simulink model "ofdm_txrx_supermimo_coded"  (Simulink 7.1)
settings: Solver=VariableStepDiscrete  SolverMode=SingleTasking  StartTime=0.0  StopTime=2*simOnly_simLength  RelTol=1e-3
PostLoadFcn:
  | ofdm_rx_supermimo_init
InitFcn:
  | ofdm_rx_supermimo_init
StartFcn:
  | ofdm_rx_supermimo_init
values from neighbouring files (symbols this model uses):
  Action0_Config = 0* 31*2^18 + 0*2^24 + PHY_AUTORESPONSE_REQ_GOODHDR + PHY_AUTORESPONSE_REQ_GOODPKT + PHY_AUTORESPONSE_REQ_FLAGA    [ofdm_tx_supermimo_init.m]
  Action1_Config = 0* 62*2^18 + PHY_AUTORESPONSE_REQ_GOODHDR + PHY_AUTORESPONSE_REQ_GOODPKT    [ofdm_tx_supermimo_init.m]
  Action2_Config = 0    [ofdm_tx_supermimo_init.m]
  Action3_Config = 0    [ofdm_tx_supermimo_init.m]
  Action4_Config = 0    [ofdm_tx_supermimo_init.m]
  Action5_Config = 0    [ofdm_tx_supermimo_init.m]
  ActionID_AFTransmit = 31    [ofdm_tx_supermimo_init.m]
  ActionID_Disabled = 0    [ofdm_tx_supermimo_init.m]
  ActionID_SetFlagA = 62    [ofdm_tx_supermimo_init.m]
  ActionID_SetFlagB = 61    [ofdm_tx_supermimo_init.m]
  BER_RAM_init_size = 2048    [ofdm_tx_supermimo_init.m]
  channel_h = [1 0; 1 0] * 0.3    [ofdm_tx_supermimo_init.m]
  CPLength = 16    [ofdm_tx_supermimo_init.m]
  FEC_Config = fec_coding_en*1 + fec_soft_dec*2 + fec_qpsk_scl*2^4 + fec_16qam_scl*2^8    (= 4195)    [ofdm_tx_supermimo_init.m]
  longCorr_coef_i = [-4*real(LTS) 0]    [ofdm_rx_supermimo_init.m]
  longCorr_coef_q = [4*imag(LTS) 0]    [ofdm_rx_supermimo_init.m]
  Match0_Config = 0    [ofdm_tx_supermimo_init.m]
  Match1_Config = 0    [ofdm_tx_supermimo_init.m]
  Match2_Config = 0    [ofdm_tx_supermimo_init.m]
  Match3_Config = 0    [ofdm_tx_supermimo_init.m]
  Match4_Config = 0    [ofdm_tx_supermimo_init.m]
  Match5_Config = 0    [ofdm_tx_supermimo_init.m]
  Match6_Config = 0    [ofdm_tx_supermimo_init.m]
  Match7_Config = 0    [ofdm_tx_supermimo_init.m]
  max_CP_length = 16    [ofdm_tx_supermimo_init.m]
  max_num_baseRateSymbols = 31    [ofdm_tx_supermimo_init.m]
  max_num_subcarriers = 64    [ofdm_tx_supermimo_init.m]
  max_num_trainingSymbols = 15    [ofdm_tx_supermimo_init.m]
  max_numBytes = 16384    [ofdm_tx_supermimo_init.m]
  max_OFDM_symbols = 1023    [ofdm_tx_supermimo_init.m]
  modConstellation_qam16 = .75*[1 3 -1 -3]./3    [ofdm_tx_supermimo_init.m]
  modConstellation_qam64 = 0.875*[3 1 5 7 -3 -1 -5 -7]./7    [ofdm_tx_supermimo_init.m]
  modConstellation_qpsk = [1 -1]./sqrt(2)    [ofdm_tx_supermimo_init.m]
  numBaseRateSymbols = numBaseRateSymbols *2    [ofdm_tx_supermimo_init.m]
  numSubcarriers = 64    [ofdm_tx_supermimo_init.m]
  numTrainingSymbols = 2    [ofdm_tx_supermimo_init.m]
  pilot_indicies = 7 + ( (64-21) * 2^8) + ( (64-7) * 2^16) + (21 * 2^24)    (= 356068103)    [ofdm_tx_supermimo_init.m]
  pilotValues = hex2dec('A57D') + (2^16 * hex2dec('5A82'))    [ofdm_tx_supermimo_init.m]
  pktByteNums = numHeaderBytes +  				byteIndex_numPayloadBytes(1)*2^8 +  				byteIndex_numPayloadBytes(2)*2^16 +  				byteIndex_simpleDynModMasks * 2^24    [ofdm_rx_supermimo_init.m]
  PktDet_Delay = 58+32 +      Symbol_Timing_Offset*2^7 +      0*2^16 +      (117* 2^24)    (= 1962935642)    [ofdm_rx_supermimo_init.m]
  pktTiming_count_payloadStart = pktTiming_count_lsStart + pktTiming_ls_num*pktTiming_ls_length    [ofdm_rx_supermimo_init.m]
  preamble = preamble_scale*[shortsyms_10 longsyms_2]    [ofdm_tx_supermimo_init.m]
  preamble_ROM = preamble_I_ROM + preamble_Q_ROM*2^16    [ofdm_tx_supermimo_init.m]
  RAM_init_size = max_numBytes    (= 16384)    [ofdm_tx_supermimo_init.m]
  reg_AF_Tx_Blanking = (320+80) + ( (320+80+79)*2^16)    (= 31392144)    [ofdm_rx_supermimo_init.m]
  reg_AF_Tx_Scaling = 2944    [ofdm_rx_supermimo_init.m]
  reg_Interrupt_PktBuf_Control = reg_InterruptControl +  	1 * 2^16 +  	0 * 2^24    (= 65536)    [ofdm_rx_supermimo_init.m]
  reg_PilotCalcParams = (4 * 2^16) + (minPilotChanMag)    (= 262184.96)    [ofdm_rx_supermimo_init.m]
  regRx_chanEst_minMag = ((0.5)*2^15) + ((0.5 * 2^15)*2^16)    (= 1073758208)    [ofdm_rx_supermimo_init.m]
  regRx_coarseCalcCorrection = round((1.3e-3 - 1.2982e-3)*2^32)    [ofdm_rx_supermimo_init.m]
  regRx_pilotCalcCorrection = round(1.00115 * 2^31)    [ofdm_rx_supermimo_init.m]
  regRx_preCFOoptions = 0 * 2^0 +  	1 * 2^1 +  	0    (= 2)    [ofdm_rx_supermimo_init.m]
  rx_controlBits = 1 * 2  		 + 1 * 4  		 + 0 * 8  		 + 1 * 16 * rx_SISO_Mode  		 + 1 * 32  		 + 1 * 64  		 + 1 * 128  		 + 1 * 256  		 + 1 * 512  		 + 1 * 1024  		 + 1 * 4096  		 + 0 * 16384  		 + 0 * 32768  		 + 1 * 65536  		 + 0 * 131072  		 + 0 * 262144  		 + 1 * 524288  		 + 1 * 1048576 * tx_Alamouti_Mode  		 + 0 * 2097152           + 1 * 33554432  		 + 0 * 4194304  		 + 0 * 8388608  		 + 0 * 16777216  		 + 1 *  ...(+49 chars)    [ofdm_rx_supermimo_init.m]
  Rx_PktDet_LongCorr_Params = LongCorrCounterLoad + 0*2^8 + 0*2^16 + 255*2^24    (= 4278190325)    [ofdm_rx_supermimo_init.m]
  Rx_PktDet_LongCorrThresholds = 8000    [ofdm_rx_supermimo_init.m]
  rx_postEq_scaling = round(rxScaling*2^11) * (1 + 2^16)    [ofdm_rx_supermimo_init.m]
  RxDataScrambling_Seq = [40 198 78 63 82 173 102 245 48 111 172 115 147 230 216 93 72 65 62 2 205 242 122 90 128 83 105 97 73 10 5 252]    [ofdm_tx_supermimo_init.m]
  RxReg_FixedPktLen = 0    [ofdm_rx_supermimo_init.m]
  rxReg_pktDetCorr = 2^00 * (floor(0.7*128)) +  	2^08 * (300) +  	0    [ofdm_rx_supermimo_init.m]
  shortSymbol_time = shortSymbol_time(1:16).'    [ofdm_tx_supermimo_init.m]
  simOnly_numSamples = length(preamble)+( (numSubcarriers+CPLength)*(numTrainingSymbols + numBaseRateSymbols + numFullRateSymbols/2) )    [ofdm_tx_supermimo_init.m]
  train = [train train]    [ofdm_tx_supermimo_init.m]
  tx_controlBits = (antB_preambleShift * 2^4) + (2^1 * tx_Alamouti_Mode) +  	(2^0 * tx_SISO_Mode) + 4*0 + 8*1 + 0*256 + 512*0 + 1024*0 +  	0*hex2dec('1000') + 0*hex2dec('2000') + 0*hex2dec('4000') +  	1*hex2dec('8000') + 1*hex2dec('10000') + hex2dec('20000') +  	0*hex2dec('100000')    [ofdm_tx_supermimo_init.m]
  tx_delays = (50*2^0) + (0*2^8) + (30*2^12)    (= 122930)    [ofdm_tx_supermimo_init.m]
  tx_scaling = (3072) + (12288 * 2^16)    (= 805309440)    [ofdm_tx_supermimo_init.m]
  TxDataScrambling_Seq = [40 198 78 63 82 173 102 245 48 111 172 115 147 230 216 93 72 65 62 2 205 242 122 90 128 83 105 97 73 10 5 252]    [ofdm_tx_supermimo_init.m]
  txReg_symbolCounts = (2^8 *numBaseRateSymbols ) + numTrainingSymbols    [ofdm_tx_supermimo_init.m]
  TxRx_FFTScaling = (3 * 2^0) +  	(2 * 2^2) +  	(1 * 2^4) +  	(1 * 2^6) +  	(1 * 2^8) +  	(0 * 2^10)    (= 347)    [ofdm_tx_supermimo_init.m]
undefined symbols (not defined in the file or in its folder): AntA, AntB, BaseRate, Down, gcbh, numBuffs, numBytes, Up, xlBlockLoadCallback, xlGetSimulinkPeriod, xlipgetpartsetting
libraries: xbsIndex_r4 x6000, simulink x4, dspsigops x4, commchan3 x2, dspsrcs4 x2, commrflib2 x2
(part 7 of 63)

== system ofdm_txrx_supermimo_coded/OFDM Rx MIMO/Coarse Freq Correction ==
  Reset : Inport  OutDataType=sfix(16)  OutScaling=2^0
  PktDet : Inport  Port=2  OutDataType=sfix(16)  OutScaling=2^0
  AntA I : Inport  Port=3  OutDataType=sfix(16)  OutScaling=2^0
  AntA Q : Inport  Port=4  OutDataType=sfix(16)  OutScaling=2^0
  AntB I : Inport  Port=5  OutDataType=sfix(16)  OutScaling=2^0
  AntB Q : Inport  Port=6  OutDataType=sfix(16)  OutScaling=2^0
  A*B : Subsystem
  A*B1 : Subsystem
  Bus Creator1 : BusCreator  Inputs=2
  CFO : Scope  NumInputPorts=5
      settings: TimeRange=14608  YMin=-0.25~-0.25~-1~-1~0  YMax=0.2~0.25~1~1~1  SaveName=ScopeData26
  Coarse CFO Calc : Subsystem
  Complex to Magnitude-Angle : ComplexToMagnitudeAngle
  Complex to Real-Imag : ComplexToRealImag
  Convert{,1..3} (4 alike) : xbsIndex_r4/Convert  arith_type="Signed  (2's comp)"  n_bits=16  bin_pt=15  quantization=Truncate  overflow=Flag as error  latency=0  dbl_ovrd=off  pipeline=off  block_type=convert
      settings: xl_area=[0 0 0 0 0 0 0]  block_version=VER_STRING_GOES_HERE
  DDS : Subsystem
  Gateway Out{,1..3} (4 alike) : xbsIndex_r4/Gateway Out  hdl_port=off  timing_constraint=None  locs_specified=off  LOCs={}  block_type=gatewayout
      settings: infoedit="Gateway out block.  Converts Xilinx fixed point inputs into ouputs of type Simulink integer, double, or fixed point.<P><P>Hardware notes:  In hardware these blocks become top level output ports or are discarded, depending on how they are configured."  xl_area=[0 0 0 0 0 0 0]  block_version=VER_STRING_GOES_HERE
  Mux2 : Mux  Inputs=2
  PreSpin CFO Sel : Subsystem
  Product : Product  OutDataType=sfix(16)  OutScaling=2^0  RndMeth=Zero
  Real-Imag to Complex : RealImagToComplex
  "AntA I " : Outport  OutDataType=sfix(16)  OutScaling=2^0
  "AntA Q " : Outport  Port=2  OutDataType=sfix(16)  OutScaling=2^0
  "AntB I " : Outport  Port=3  OutDataType=sfix(16)  OutScaling=2^0
  "AntB Q " : Outport  Port=4  OutDataType=sfix(16)  OutScaling=2^0
  tag scope: CFO_PhaseInc=global
  wires:
  A*B/1(Re) -> Convert/1
  A*B/2(Im1) -> Convert1/1
  A*B1/1(Re) -> Convert2/1
  A*B1/2(Im1) -> Convert3/1
  DDS/1(Q) -> Gateway Out/1, A*B1/2(Im[B]), A*B/3(Im[B])
  DDS/2(I) -> Gateway Out1/1, A*B1/1(Re[B]), A*B/4(Re[B])
  Gateway Out1/1 -> Mux2/1, Real-Imag to Complex/1
  Gateway Out/1 -> Mux2/2, Real-Imag to Complex/2
  Mux2/1 -> CFO/3
  Bus Creator1/1 -> CFO/4
  [freqOffset] -> Complex to Real-Imag/1, Product/2
  Complex to Real-Imag/1 -> Bus Creator1/1
  Complex to Real-Imag/2 -> Bus Creator1/2
  Gateway Out2/1 -> CFO/2
  Gateway Out3/1 -> CFO/1
  AntB I/1 -> A*B1/3(Re[A])
  AntB Q/1 -> A*B1/4(Im[A])
  AntA I/1 -> A*B/1(Re[A]), Coarse CFO Calc/1(I In)
  AntA Q/1 -> A*B/2(Im[A]), Coarse CFO Calc/2(Q In)
  Convert/1 -> "AntA I "/1
  Convert1/1 -> "AntA Q "/1
  Real-Imag to Complex/1 -> Product/1
  Product/1 -> Complex to Magnitude-Angle/1
  Complex to Magnitude-Angle/2 -> CFO/5
  Convert2/1 -> "AntB I "/1
  Convert3/1 -> "AntB Q "/1
  Reset/1 -> Coarse CFO Calc/4(Reset), DDS/3(Rst), PreSpin CFO Sel/1(Rst)
  PktDet/1 -> Gateway Out2/1, Coarse CFO Calc/3(RxPkt Active)
  Coarse CFO Calc/1(Phase Inc) -> Gateway Out3/1, [CFO_PhaseInc], DDS/2(Rx_CoarseEst)
  PreSpin CFO Sel/1(Tx_PhaseInc) -> DDS/1(Tx_PhaseInc)

== system ofdm_txrx_supermimo_coded/OFDM Rx MIMO/Equalizer & Packetizer ==
  Payload : Inport  OutDataType=sfix(16)  OutScaling=2^0
  Y_I : Inport  Port=2  OutDataType=sfix(16)  OutScaling=2^0
  Y_Q : Inport  Port=3  OutDataType=sfix(16)  OutScaling=2^0
  H-I : Inport  Port=4  OutDataType=sfix(16)  OutScaling=2^0
  H-Q : Inport  Port=5  OutDataType=sfix(16)  OutScaling=2^0
  SubCar Index : Inport  Port=6  OutDataType=sfix(16)  OutScaling=2^0
  Sym Index : Inport  Port=7  OutDataType=sfix(16)  OutScaling=2^0
  Vin : Inport  Port=8  OutDataType=sfix(16)  OutScaling=2^0
  Phases I : Inport  Port=9  OutDataType=sfix(16)  OutScaling=2^0
  Phases Q : Inport  Port=10  OutDataType=sfix(16)  OutScaling=2^0
  Convert4 : xbsIndex_r4/Convert  arith_type="Signed  (2's comp)"  n_bits=14  bin_pt=13  quantization=Truncate  overflow=Wrap  latency=0  dbl_ovrd=off  pipeline=off  block_type=convert
      settings: xl_area=[0, 0, 0, 0, 0, 0, 0]  block_version=VER_STRING_GOES_HERE
  Convert5 : xbsIndex_r4/Convert  arith_type="Signed  (2's comp)"  n_bits=14  bin_pt=13  quantization=Truncate  overflow=Wrap  latency=0  dbl_ovrd=off  pipeline=off  block_type=convert
      settings: xl_area=[0, 0, 0, 0, 0, 0, 0]  block_version=VER_STRING_GOES_HERE
  Eq : Scope  NumInputPorts=7
      settings: YMin=-0.5~-0.5~0~0~15~0.95~-1  YMax=0.5~0.5~70~1~20~1.05~1  SaveName=ScopeData14
  Equalizer : Subsystem
  Packet_Constructor : Subsystem
  Real-Imag to Complex : RealImagToComplex
  Rx_debug_EQ_I : xbsIndex_r4/Gateway Out  hdl_port=on  timing_constraint=None  locs_specified=off  LOCs={}  block_type=gatewayout
      settings: infoedit="Gateway out block.  Converts Xilinx fixed point inputs into ouputs of type Simulink integer, double, or fixed point.<P><P>Hardware notes:  In hardware these blocks become top level output ports or are discarded, depending on how they are configured."  xl_area=[0 0 0 0 1 0 0]  block_version=VER_STRING_GOES_HERE
  Rx_debug_EQ_Q : xbsIndex_r4/Gateway Out  hdl_port=on  timing_constraint=None  locs_specified=off  LOCs={}  block_type=gatewayout
      settings: infoedit="Gateway out block.  Converts Xilinx fixed point inputs into ouputs of type Simulink integer, double, or fixed point.<P><P>Hardware notes:  In hardware these blocks become top level output ports or are discarded, depending on how they are configured."  xl_area=[0 0 0 0 1 0 0]  block_version=VER_STRING_GOES_HERE
  Scaling : Subsystem
  Up Sample1 : xbsIndex_r4/Up Sample  sample_ratio=2  copy_samples=on  dbl_ovrd=off  block_type=usamp
      settings: infoedit="Up samples input data.  Inserted values  can be zeros or copies of the most recent input sample.<P><P>Hardware notes: No hardware is needed if inserted values are copies of the input sample; otherwise, a mux and single bit flip-flop are used."  block_version=8.2.02
  Up Sample2 : xbsIndex_r4/Up Sample  sample_ratio=2  copy_samples=on  dbl_ovrd=off  block_type=usamp
      settings: infoedit="Up samples input data.  Inserted values  can be zeros or copies of the most recent input sample.<P><P>Hardware notes: No hardware is needed if inserted values are copies of the input sample; otherwise, a mux and single bit flip-flop are used."  block_version=8.2.02
  Zero-Order Hold : ZeroOrderHold
  Zero-Order Hold1 : ZeroOrderHold
  done{1..6} (6 alike) : xbsIndex_r4/Gateway Out  hdl_port=off  timing_constraint=None  locs_specified=off  LOCs={}  block_type=gatewayout
      settings: infoedit="Gateway out block.  Converts Xilinx fixed point inputs into ouputs of type Simulink integer, double, or fixed point.<P><P>Hardware notes:  In hardware these blocks become top level output ports or are discarded, depending on how they are configured."  xl_area=[0 0 0 0 1 0 0]  block_version=VER_STRING_GOES_HERE
  posedge : Subsystem
  done : Outport  OutDataType=sfix(16)  OutScaling=2^0
  wires:
  SubCar Index/1 -> Equalizer/9(SubCar Index)
  Sym Index/1 -> Equalizer/8(Sym Index)
  Scaling/1(I) -> Packet_Constructor/1(I), Convert4/1
  Scaling/2(Q) -> Packet_Constructor/2(Q), Convert5/1
  Scaling/3(" Xk_index") -> Packet_Constructor/3(Xk_index)
  Scaling/4(Vout) -> Packet_Constructor/4(Vin)
  Scaling/5(" Sym Index") -> Packet_Constructor/5(Sym Index)
  Packet_Constructor/1(done) -> done/1
  H-I/1 -> Equalizer/5(H-I)
  H-Q/1 -> Equalizer/6(H-Q)
  Vin/1 -> Equalizer/7(Vin)
  Y_I/1 -> Equalizer/3(Y_I)
  Y_Q/1 -> Equalizer/4(Y_Q)
  Payload/1 -> posedge/1(D), done6/1
  posedge/1(Q) -> Packet_Constructor/6(Start)
  Equalizer/1(I-eq) -> Scaling/1(Eq-I), done4/1
  Equalizer/2(Q-eq) -> Scaling/2(Eq-Q), done5/1
  Equalizer/3(" Xk_index") -> Scaling/3(Xk_index), done1/1
  Equalizer/4(Vout) -> Scaling/4(Vin), done2/1
  Equalizer/5(" Sym Index") -> Scaling/5(Sym Index), done3/1
  Up Sample2/1 -> Rx_debug_EQ_Q/1
  Up Sample1/1 -> Rx_debug_EQ_I/1
  Convert5/1 -> Up Sample2/1
  Convert4/1 -> Up Sample1/1
  Phases I/1 -> Equalizer/1(Phases I)
  Phases Q/1 -> Equalizer/2(Phases Q)
  Rx_debug_EQ_I/1 -> Zero-Order Hold/1
  Rx_debug_EQ_Q/1 -> Zero-Order Hold1/1
  Real-Imag to Complex/1 -> ?
  Zero-Order Hold/1 -> Real-Imag to Complex/1
  Zero-Order Hold1/1 -> Real-Imag to Complex/2
  done4/1 -> Eq/1
  done5/1 -> Eq/2
  done1/1 -> Eq/3
  done2/1 -> Eq/4
  done3/1 -> Eq/5
  done6/1 -> Eq/6

== system ofdm_txrx_supermimo_coded/OFDM Rx MIMO/FFT & Chan Est ==
  note: Payload->WE latency was 1 - why?
  Payload : Inport  OutDataType=sfix(16)  OutScaling=2^0
  AntA I : Inport  Port=2  OutDataType=sfix(16)  OutScaling=2^0
  AntA Q : Inport  Port=3  OutDataType=sfix(16)  OutScaling=2^0
  AntB I : Inport  Port=4  OutDataType=sfix(16)  OutScaling=2^0
  AntB Q : Inport  Port=5  OutDataType=sfix(16)  OutScaling=2^0
  Addr Gen1 : Subsystem
  Addr_Generator : Subsystem
  Assert : xbsIndex_r4/Assert  Orientation=left  assert_type=off  type_source=Explicitly  arith_type="Signed  (2's comp)"  n_bits=16  bin_pt=14  assert_rate=on  rate_source=Explicitly  period=1  output_port=on  block_type=assert
      settings: infoedit=Asserts a user-defined sample rate and/or type on a signal.<P><P>Hardware notes: In hardware this block costs nothing.  block_version=VER_STRING_GOES_HERE
  Channel Estimation & Pilot Processing : Subsystem
  Delay{1..4} (4 alike) : xbsIndex_r4/Delay  en=off  latency=1  dbl_ovrd=off  reg_retiming=off  block_type=delay
      settings: infoedit=Hardware notes: A delay line is a chain, each link of which is an SRL16 followed by a flip-flop. If register retiming is enabled, the delay line is a chain of flip-flops.  block_version=VER_STRING_GOES_HERE
  Down Sample : xbsIndex_r4/Down Sample  Orientation=left  sample_ratio=2  sample_phase=First Value of Frame  en=off  latency=0  dbl_ovrd=off  block_type=dsamp
      settings: block_version=VER_STRING_GOES_HERE
  FFT : Subsystem
  Input Buffers : Subsystem
  Inverter2 : xbsIndex_r4/Inverter  en=off  latency=0  dbl_ovrd=off  block_type=inv
      settings: block_version=VER_STRING_GOES_HERE
  Output Buffers : Subsystem
  Symbol Counter : Subsystem
  Y_I : Outport  OutDataType=sfix(16)  OutScaling=2^0
  Y_Q : Outport  Port=2  OutDataType=sfix(16)  OutScaling=2^0
  H-I : Outport  Port=3  OutDataType=sfix(16)  OutScaling=2^0
  H-Q : Outport  Port=4  OutDataType=sfix(16)  OutScaling=2^0
  SubCar Index : Outport  Port=5  OutDataType=sfix(16)  OutScaling=2^0
  Sym Index : Outport  Port=6  OutDataType=sfix(16)  OutScaling=2^0
  Vout : Outport  Port=7  OutDataType=sfix(16)  OutScaling=2^0
  Phase I : Outport  Port=8  OutDataType=sfix(16)  OutScaling=2^0
  Phase Q : Outport  Port=9  OutDataType=sfix(16)  OutScaling=2^0
  tag scope: FFT_I=global
  wires:
  Delay1/1 -> Input Buffers/5(We)
  FFT/5(Done) -> Symbol Counter/1(FFT Done), Addr Gen1/1(FFT Done)
  Addr_Generator/3(Ant Sel) -> Input Buffers/8(Ant Sel)
  Assert/1 -> Addr_Generator/2(FFT Xn_index)
  AntB Q/1 -> Input Buffers/4(AntB Q)
  AntB I/1 -> Input Buffers/3(AntB I)
  AntA Q/1 -> Input Buffers/2(AntA Q)
  AntA I/1 -> Input Buffers/1(AntA I)
  Payload/1 -> Delay1/1, Addr_Generator/1(Payload), Symbol Counter/2(Payload), Addr Gen1/2(Payload), Inverter2/1
  Addr_Generator/2(Read Addr) -> Input Buffers/7(Rd Addr)
  Addr_Generator/5(FFT Start) -> FFT/3(Start)
  Input Buffers/2(" Q") -> FFT/2(xn_Q)
  Input Buffers/1(" I") -> FFT/1(xn_I)
  Addr_Generator/1(Write Addr) -> Input Buffers/6(Wr Addr)
  Delay4/1 -> SubCar Index/1
  Delay2/1 -> Vout/1
  Output Buffers/2(" Q") -> Y_Q/1
  Channel Estimation & Pilot Processing/2(H-Q) -> H-Q/1
  Channel Estimation & Pilot Processing/1(H-I) -> H-I/1
  Output Buffers/1(" I") -> Y_I/1
  Channel Estimation & Pilot Processing/5(Est Vout) -> Down Sample/1
  Symbol Counter/1(Sym Index) -> Channel Estimation & Pilot Processing/5(Sym Index), Output Buffers/5(SymIndex)
  FFT/4(Vout) -> Channel Estimation & Pilot Processing/4(Vin), Output Buffers/4(FFT Vout)
  FFT/3(xk_Index) -> Channel Estimation & Pilot Processing/3(Xk Index), Output Buffers/3(Wr Addr)
  FFT/2(xk_Q) -> Output Buffers/2(Q), Channel Estimation & Pilot Processing/2(Q)
  FFT/1(xk_I) -> Channel Estimation & Pilot Processing/1(I), Output Buffers/1(I), [FFT_I]
  FFT/6(xn_Index) -> Assert/1
  Down Sample/1 -> Addr Gen1/3(NotTraining)
  Addr Gen1/2(Rd Addr) -> Output Buffers/6(Rd Addr), Channel Estimation & Pilot Processing/6(Est Index), Delay4/1
  Addr Gen1/3(Vout) -> Delay2/1
  Delay3/1 -> Sym Index/1
  Addr Gen1/1(SymIndex_out) -> Delay3/1
  Addr_Generator/4(Wr Slot) -> Input Buffers/9(Wr Slot)
  Channel Estimation & Pilot Processing/3(Phase I) -> Phase I/1
  Channel Estimation & Pilot Processing/4(Phase Q) -> Phase Q/1
  Inverter2/1 -> Channel Estimation & Pilot Processing/7(Rst)

== system ofdm_txrx_supermimo_coded/OFDM Rx MIMO/Long Corr HP ==
  note: This block was originally designed by Dr. Chris Dick (Cheif DSP Scientist at Xilinx)
      | It's been modified since to run at 4x (instead of 5x, as in the original OFDM designs)
      |
      | This block runs a 64-point 1-bit by 1-bit correlation per clock cycle. Using 1-bit per
      | operand allows just add/sub operations instead of actual multiplicaitons,
      | which would be hugely expensive. This degrades performance a little,
      | but the LTS have good enough autocorrelation properties that there is still
      | plenth of margin using this scheme.
  I In : Inport  OutDataType=sfix(16)  OutScaling=2^0
  Q In : Inport  Port=2  OutDataType=sfix(16)  OutScaling=2^0
  AddSub : xbsIndex_r4/AddSub  mode=Subtraction  use_carryin=off  use_carryout=off  en=off  latency=0  precision=Full  arith_type=Unsigned  n_bits=8  bin_pt=2  quantization=Truncate  overflow=Wrap  dbl_ovrd=off  use_behavioral_HDL=on  pipelined=off  use_rpm=off  hw_selection=Fabric  block_type=addsub
      settings: xl_area=[5 0 0 8 0 0 0]  block_version=VER_STRING_GOES_HERE
  AddSub1 : xbsIndex_r4/AddSub  mode=Addition  use_carryin=off  use_carryout=off  en=off  latency=0  precision=Full  arith_type=Unsigned  n_bits=8  bin_pt=2  quantization=Truncate  overflow=Wrap  dbl_ovrd=off  use_behavioral_HDL=on  pipelined=off  use_rpm=off  hw_selection=Fabric  block_type=addsub
      settings: xl_area=[5 0 0 8 0 0 0]  block_version=VER_STRING_GOES_HERE
  AddSub2 : xbsIndex_r4/AddSub  mode=Addition  use_carryin=off  use_carryout=off  en=off  latency=0  precision=Full  arith_type=Unsigned  n_bits=8  bin_pt=2  quantization=Truncate  overflow=Wrap  dbl_ovrd=off  use_behavioral_HDL=on  pipelined=off  use_rpm=off  hw_selection=Fabric  block_type=addsub
      settings: xl_area=[10 0 0 18 0 0 0]  block_version=VER_STRING_GOES_HERE
  C1 : Subsystem  mask{ns=0, N=4, h=longCorr_coef_i, Ts_In=4}
      mask prompts: ns "Num sub-correlator"; N "Coefficients/correlator"; h "Coefficients"; Ts_In "Input sample period"
  C2 : Subsystem  mask{ns=0, N=4, h=longCorr_coef_q, Ts_In=4}
      mask prompts: ns "Num sub-correlator"; N "Coefficients/correlator"; h "Coefficients"; Ts_In "Input sample period"
  C3 : Subsystem  mask{ns=0, N=4, h=longCorr_coef_i, Ts_In=4}
      mask prompts: ns "Num sub-correlator"; N "Coefficients/correlator"; h "Coefficients"; Ts_In "Input sample period"
  C4 : Subsystem  mask{ns=0, N=4, h=longCorr_coef_q, Ts_In=4}
      mask prompts: ns "Num sub-correlator"; N "Coefficients/correlator"; h "Coefficients"; Ts_In "Input sample period"
  Mult : xbsIndex_r4/Mult  precision=Full  arith_type="Signed  (2's comp)"  n_bits=8  bin_pt=2  quantization=Truncate  overflow=Wrap  en=off  latency=1  dbl_ovrd=off  use_behavioral_HDL=off  use_embedded=on  opt=Speed  optimum_pipeline=off  pipeline=on  use_rpm=off  placement_style=Rectangular shape  block_type=mult
      settings: infoedit=Hardware notes: To use the internal pipeline stage of the dedicated multiplier you must select 'Pipeline for maximum performance'.  xl_area=[60 18 0 106 0 0 0]  block_version=VER_STRING_GOES_HERE
  Mult1 : xbsIndex_r4/Mult  precision=Full  arith_type="Signed  (2's comp)"  n_bits=8  bin_pt=2  quantization=Truncate  overflow=Wrap  en=off  latency=1  dbl_ovrd=off  use_behavioral_HDL=off  use_embedded=on  opt=Speed  optimum_pipeline=off  pipeline=on  use_rpm=off  placement_style=Rectangular shape  block_type=mult
      settings: infoedit=Hardware notes: To use the internal pipeline stage of the dedicated multiplier you must select 'Pipeline for maximum performance'.  xl_area=[60 18 0 106 0 0 0]  block_version=VER_STRING_GOES_HERE
  Slice : xbsIndex_r4/Slice  nbits=1  boolean_output=off  mode=Upper Bit Location + Width  bit1=0  base1=MSB of Input  bit0=0  base0=LSB of Input  dbl_ovrd=off  block_type=slice
      settings: infoedit="Extracts a given range of bits from each input sample and presents it at the output.  The output type is ordinarily unsigned with binary point at zero, but can be Boolean when the slice is one bit wide.<P><P>Hardware notes: In hardware this block costs nothing."  block_version=10.1.3
  Slice1 : xbsIndex_r4/Slice  nbits=1  boolean_output=off  mode=Upper Bit Location + Width  bit1=0  base1=MSB of Input  bit0=0  base0=LSB of Input  dbl_ovrd=off  block_type=slice
      settings: infoedit="Extracts a given range of bits from each input sample and presents it at the output.  The output type is ordinarily unsigned with binary point at zero, but can be Boolean when the slice is one bit wide.<P><P>Hardware notes: In hardware this block costs nothing."  block_version=10.1.3
  y : Outport  OutDataType=sfix(16)  OutScaling=2^0
  tag scope: Long_Corr=global
  wires:
  Slice1/1 -> C2/1(x), C3/1(x)
  Slice/1 -> C1/1(x), C4/1(x)
  C1/1(y) -> AddSub/1
  AddSub2/1 -> y/1, [Long_Corr]
  Mult1/1 -> AddSub2/2
  Mult/1 -> AddSub2/1
  AddSub1/1 -> Mult1/2, Mult1/1
  AddSub/1 -> Mult/2, Mult/1
  C3/1(y) -> AddSub1/2
  C4/1(y) -> AddSub1/1
  C2/1(y) -> AddSub/2
  Q In/1 -> Slice1/1
  I In/1 -> Slice/1

== system ofdm_txrx_supermimo_coded/OFDM Rx MIMO/Packet_Detection ==
  Pkt Active : Inport  OutDataType=sfix(16)  OutScaling=2^0
  Reset : Inport  Port=2  OutDataType=sfix(16)  OutScaling=2^0
  Payload : Inport  Port=3  OutDataType=sfix(16)  OutScaling=2^0
  Auto-Correlation Pkt Det HARD MULT : Subsystem
  Auto-Correlation Pkt Det HDL MULT : Subsystem
  Constant : xbsIndex_r4/Constant  arith_type=Boolean  const=1  n_bits=1  bin_pt=0  explicit_period=on  period=1  equ=P=C  opselect=C  inp2=PCIN>>17  opr=+  inp1=P  carry=CIN  dbl_ovrd=off  block_type=constant
  Counter1 : xbsIndex_r4/Counter  cnt_type=Free Running  cnt_to=Inf  operation=Up  start_count=0  cnt_by_val=1  arith_type=Unsigned  n_bits=32  bin_pt=0  load_pin=off  rst=off  en=on  explicit_period=off  period=4  dbl_ovrd=off  use_behavioral_HDL=off  use_rpm=off  implementation=Fabric  block_type=counter
  Ext PktDet : Subsystem
  Inverter4 : xbsIndex_r4/Inverter  en=off  latency=0  dbl_ovrd=off  block_type=inv
      settings: block_version=8.2
  Inverter7 : xbsIndex_r4/Inverter  en=off  latency=0  dbl_ovrd=off  block_type=inv
      settings: block_version=8.2
  Logical2 : xbsIndex_r4/Logical  logical_function=OR  inputs=3  en=off  latency=0  precision=Full  arith_type=Unsigned  n_bits=16  bin_pt=0  align_bp=on  dbl_ovrd=off  block_type=logical
      settings: block_version=8.2
  Logical3 : xbsIndex_r4/Logical  logical_function=AND  inputs=2  en=off  latency=0  precision=Full  arith_type=Unsigned  n_bits=16  bin_pt=0  align_bp=on  dbl_ovrd=off  block_type=logical
      settings: block_version=8.2
  Logical4 : xbsIndex_r4/Logical  logical_function=AND  inputs=3  en=off  latency=0  precision=Full  arith_type=Unsigned  n_bits=16  bin_pt=0  align_bp=on  dbl_ovrd=off  block_type=logical
      settings: block_version=8.2
  Logical5 : xbsIndex_r4/Logical  logical_function=OR  inputs=2  en=off  latency=0  precision=Full  arith_type=Unsigned  n_bits=16  bin_pt=0  align_bp=on  dbl_ovrd=off  block_type=logical
      settings: block_version=8.2
  Logical6 : xbsIndex_r4/Logical  logical_function=AND  inputs=2  en=off  latency=0  precision=Full  arith_type=Unsigned  n_bits=16  bin_pt=0  align_bp=on  dbl_ovrd=off  block_type=logical
      settings: block_version=8.2
  Posedge : Subsystem
  RSSI-based PktDet : Subsystem
  Register1 : xbsIndex_r4/Register  init=0  rst=off  en=off  dbl_ovrd=off  block_type=register
  Register2 : xbsIndex_r4/Register  init=0  rst=off  en=off  dbl_ovrd=off  block_type=register
  Up Sample1 : xbsIndex_r4/Up Sample  sample_ratio=4  copy_samples=on  dbl_ovrd=off  block_type=usamp
      settings: infoedit="Up samples input data.  Inserted values  can be zeros or copies of the most recent input sample.<P><P>Hardware notes: No hardware is needed if inserted values are copies of the input sample; otherwise, a mux and single bit flip-flop are used."  block_version=8.2
  debug_pktDetAutoCorr : xbsIndex_r4/Gateway Out  hdl_port=on  timing_constraint=None  locs_specified=off  LOCs={}  block_type=gatewayout
      settings: infoedit="Gateway out block.  Converts Xilinx fixed point inputs into ouputs of type Simulink integer, double, or fixed point.<P><P>Hardware notes:  In hardware these blocks become top level output ports or are discarded, depending on how they are configured."  block_version=VER_STRING_GOES_HERE
  debug_pktDetRSSI : xbsIndex_r4/Gateway Out  hdl_port=on  timing_constraint=None  locs_specified=off  LOCs={}  block_type=gatewayout
      settings: infoedit="Gateway out block.  Converts Xilinx fixed point inputs into ouputs of type Simulink integer, double, or fixed point.<P><P>Hardware notes:  In hardware these blocks become top level output ports or are discarded, depending on how they are configured."  block_version=VER_STRING_GOES_HERE
  pktdet : xbsIndex_r4/Gateway Out  hdl_port=on  timing_constraint=None  locs_specified=off  LOCs={}  block_type=gatewayout
      settings: infoedit="Gateway out block.  Converts Xilinx fixed point inputs into ouputs of type Simulink integer, double, or fixed point.<P><P>Hardware notes:  In hardware these blocks become top level output ports or are discarded, depending on how they are configured."  block_version=VER_STRING_GOES_HERE
  Pkt Det : Outport  OutDataType=sfix(16)  OutScaling=2^0
  tag scope: pktDet=global, pktDet_corr=global, pktDet_rssi=global, regRx_pktDetEventCount=global, regRx_pktDetEventCount_en=global
  wires:
  [AntA_ADC_I_Filt] -> Auto-Correlation Pkt Det HARD MULT/1(AntA I), Auto-Correlation Pkt Det HDL MULT/1(AntA I)
  [AntA_ADC_Q_Filt] -> Auto-Correlation Pkt Det HARD MULT/2(AntA Q), Auto-Correlation Pkt Det HDL MULT/2(AntA Q)
  Reset/1 -> RSSI-based PktDet/3(PktDet Reset), Inverter7/1
  Pkt Active/1 -> Logical5/1
  [Tx_Running] -> Logical5/2
  Posedge/1(Q) -> Counter1/1
  Counter1/1 -> Up Sample1/1
  Constant/1 -> [regRx_pktDetEventCount_en]
  Up Sample1/1 -> [regRx_pktDetEventCount]
  Register1/1 -> debug_pktDetRSSI/1
  Inverter4/1 -> Logical4/2
  Inverter7/1 -> Logical4/3
  [regPktDet_IgnoreDetection] -> Inverter4/1
  Logical2/1 -> Logical4/1
  Payload/1 -> RSSI-based PktDet/1(Payload)
  Logical5/1 -> RSSI-based PktDet/2(Force Busy)
  Ext PktDet/1(Det) -> Logical2/1
  Auto-Correlation Pkt Det HARD MULT/1(PktDet) -> Logical3/2
  [regPktDet_enableAutoCorrDet] -> Logical3/1
  RSSI-based PktDet/1(PktDet) -> Logical6/2
  [regPktDet_enableRSSIdet] -> Logical6/1
  Logical6/1 -> Logical2/3, Register1/1, [pktDet_rssi]
  Logical3/1 -> Logical2/2, Register2/1, [pktDet_corr]
  Logical4/1 -> Pkt Det/1, Posedge/1(D), [pktDet], pktdet/1
  Register2/1 -> debug_pktDetAutoCorr/1

== system ofdm_txrx_supermimo_coded/OFDM Rx MIMO/Packet_Timing_Control ==
  Pkt Det : Inport  OutDataType=sfix(16)  OutScaling=2^0
  Long CorrA : Inport  Port=2  OutDataType=sfix(16)  OutScaling=2^0
  Done : Inport  Port=3  OutDataType=sfix(16)  OutScaling=2^0
  ASR : xbsIndex_r4/Addressable Shift Register  infer_latency=on  depth=2  initVector=[0]  en=off  dbl_ovrd=off  use_behavioral_HDL=off  use_rpm=off  block_type=addrsr
      settings: infoedit="Delay of  configurable length.  Any element  in the delay     line   can    be   addressed   and   driven    onto   the   output     port.<P><P>Hardware  notes: Implemented  using SRL16s.     If Virtex-4, Virtex-II or Spartan-3 devices are used, multiple SRLC16s are cascaded together."  xl_area=[13 0 0 25 0 0 0]  block_version=VER_STRING_GOES_HERE
  Assert : xbsIndex_r4/Assert  Orientation=left  assert_type=on  type_source=Explicitly  arith_type=Boolean  n_bits=16  bin_pt=14  assert_rate=on  rate_source=Explicitly  period=4  output_port=on  block_type=assert
      settings: infoedit=Asserts a user-defined sample rate and/or type on a signal.<P><P>Hardware notes: In hardware this block costs nothing.  block_version=VER_STRING_GOES_HERE
  Constant1 : xbsIndex_r4/Constant  arith_type=Boolean  const=0  n_bits=1  bin_pt=0  explicit_period=off  period=1  equ=P=C  opselect=C  inp2=PCIN>>17  opr=+  inp1=P  carry=CIN  dbl_ovrd=off  block_type=constant
      settings: block_version=VER_STRING_GOES_HERE
  Constant11 : xbsIndex_r4/Constant  arith_type=Unsigned  const=pktTiming_count_payloadStart-2  n_bits=ceil(log2(pktTiming_count_payloadStart))  bin_pt=0  explicit_period=off  period=1  equ=P=C  opselect=C  inp2=PCIN>>17  opr=+  inp1=P  carry=CIN  dbl_ovrd=off  block_type=constant
      settings: block_version=VER_STRING_GOES_HERE
  Constant6 : xbsIndex_r4/Constant  arith_type=Unsigned  const=pktTiming_count_payloadStart  n_bits=ceil(log2(pktTiming_count_payloadStart))+1  bin_pt=0  explicit_period=off  period=1  equ=P=C  opselect=C  inp2=PCIN>>17  opr=+  inp1=P  carry=CIN  dbl_ovrd=off  block_type=constant
      settings: block_version=VER_STRING_GOES_HERE
  Convert1 : xbsIndex_r4/Convert  arith_type=Unsigned  n_bits=1  bin_pt=0  quantization=Truncate  overflow=Wrap  latency=0  dbl_ovrd=off  pipeline=off  block_type=convert
      settings: xl_area=[0, 0, 0, 0, 0, 0, 0]  block_version=VER_STRING_GOES_HERE
  Convert2 : xbsIndex_r4/Convert  arith_type=Unsigned  n_bits=16  bin_pt=0  quantization=Truncate  overflow=Wrap  latency=0  dbl_ovrd=off  pipeline=off  block_type=convert
      settings: xl_area=[0, 0, 0, 0, 0, 0, 0]  block_version=VER_STRING_GOES_HERE
  Copy_Downsample : Subsystem
  Counter : xbsIndex_r4/Counter  cnt_type=Free Running  cnt_to=Inf  operation=Up  start_count=0  cnt_by_val=1  arith_type=Unsigned  n_bits=pktTiming_controlCounter_bits  bin_pt=0  load_pin=on  rst=on  en=on  explicit_period=off  period=1  dbl_ovrd=off  use_behavioral_HDL=off  use_rpm=on  implementation=Fabric  block_type=counter
      settings: xl_area=[6 11 0 12 0 0 0]  block_version=VER_STRING_GOES_HERE
  Disable for Interrupts : Subsystem
  Edge Det : Subsystem
  Edge Det1 : Subsystem
  Ext Reset : Subsystem  Orientation=left
  Fine_Pkt_det : Subsystem
  Inverter1 : xbsIndex_r4/Inverter  en=off  latency=0  dbl_ovrd=off  block_type=inv
      settings: xl_area=[0 0 0 0 0 0 0]  block_version=VER_STRING_GOES_HERE
  Inverter3 : xbsIndex_r4/Inverter  en=off  latency=0  dbl_ovrd=off  block_type=inv
      settings: xl_area=[0 0 0 0 0 0 0]  block_version=VER_STRING_GOES_HERE
  Logical2 : xbsIndex_r4/Logical  logical_function=AND  inputs=2  en=off  latency=0  precision=Full  arith_type=Unsigned  n_bits=8  bin_pt=2  align_bp=on  dbl_ovrd=off  block_type=logical
      settings: xl_area=[1 0 0 1 0 0 0]  block_version=VER_STRING_GOES_HERE
  Logical3 : xbsIndex_r4/Logical  logical_function=AND  inputs=2  en=off  latency=0  precision=Full  arith_type=Unsigned  n_bits=8  bin_pt=2  align_bp=on  dbl_ovrd=off  block_type=logical
      settings: xl_area=[1 0 0 1 0 0 0]  block_version=VER_STRING_GOES_HERE
  Logical4 : xbsIndex_r4/Logical  Orientation=left  logical_function=AND  inputs=2  en=off  latency=0  precision=Full  arith_type=Unsigned  n_bits=8  bin_pt=2  align_bp=on  dbl_ovrd=off  block_type=logical
      settings: xl_area=[1 0 0 1 0 0 0]  block_version=VER_STRING_GOES_HERE
  Logical5 : xbsIndex_r4/Logical  Orientation=left  logical_function=OR  inputs=6  en=off  latency=0  precision=Full  arith_type=Unsigned  n_bits=8  bin_pt=2  align_bp=on  dbl_ovrd=off  block_type=logical
      settings: xl_area=[1 0 0 1 0 0 0]  block_version=VER_STRING_GOES_HERE
  Logical6 : xbsIndex_r4/Logical  logical_function=AND  inputs=3  en=off  latency=0  precision=Full  arith_type=Unsigned  n_bits=8  bin_pt=2  align_bp=on  dbl_ovrd=off  block_type=logical
      settings: xl_area=[1 0 0 1 0 0 0]  block_version=VER_STRING_GOES_HERE
  Relational5 : xbsIndex_r4/Relational  mode=a>b  en=off  latency=0  dbl_ovrd=off  block_type=relational
      settings: xl_area=[6 0 0 11 0 0 0]  block_version=VER_STRING_GOES_HERE
  Relational6 : xbsIndex_r4/Relational  mode=a>b  en=off  latency=0  dbl_ovrd=off  block_type=relational
      settings: xl_area=[6 0 0 11 0 0 0]  block_version=VER_STRING_GOES_HERE
  Rx_PktDetReset : xbsIndex_r4/Gateway Out  hdl_port=on  timing_constraint=None  locs_specified=off  LOCs={}  block_type=gatewayout
      settings: infoedit="Gateway out block.  Converts Xilinx fixed point inputs into ouputs of type Simulink integer, double, or fixed point.<P><P>Hardware notes:  In hardware these blocks become top level output ports or are discarded, depending on how they are configured."  xl_area=[0, 0, 0, 0, 0, 0, 0]  block_version=VER_STRING_GOES_HERE
  Rx_debug_PktDone : xbsIndex_r4/Gateway Out  hdl_port=on  timing_constraint=None  locs_specified=off  LOCs={}  block_type=gatewayout
      settings: infoedit="Gateway out block.  Converts Xilinx fixed point inputs into ouputs of type Simulink integer, double, or fixed point.<P><P>Hardware notes:  In hardware these blocks become top level output ports or are discarded, depending on how they are configured."  xl_area=[0, 0, 0, 0, 0, 0, 0]  block_version=VER_STRING_GOES_HERE
  Rx_debug_payload : xbsIndex_r4/Gateway Out  hdl_port=on  timing_constraint=None  locs_specified=off  LOCs={}  block_type=gatewayout
      settings: infoedit="Gateway out block.  Converts Xilinx fixed point inputs into ouputs of type Simulink integer, double, or fixed point.<P><P>Hardware notes:  In hardware these blocks become top level output ports or are discarded, depending on how they are configured."  xl_area=[0 0 0 0 1 0 0]  block_version=VER_STRING_GOES_HERE
  S-R Latch : Subsystem
  S-R Latch1 : Subsystem
  Up Sample : xbsIndex_r4/Up Sample  sample_ratio=4  copy_samples=on  dbl_ovrd=off  block_type=usamp
      settings: infoedit="Up samples input data.  Inserted values  can be zeros or copies of the most recent input sample.<P><P>Hardware notes: No hardware is needed if inserted values are copies of the input sample; otherwise, a mux and single bit flip-flop are used."  block_version=8.2.02
  Up Sample1 : xbsIndex_r4/Up Sample  sample_ratio=2  copy_samples=on  dbl_ovrd=off  block_type=usamp
      settings: infoedit="Up samples input data.  Inserted values  can be zeros or copies of the most recent input sample.<P><P>Hardware notes: No hardware is needed if inserted values are copies of the input sample; otherwise, a mux and single bit flip-flop are used."  block_version=8.2.02
  Up Sample2 : xbsIndex_r4/Up Sample  sample_ratio=4  copy_samples=on  dbl_ovrd=off  block_type=usamp
      settings: infoedit="Up samples input data.  Inserted values  can be zeros or copies of the most recent input sample.<P><P>Hardware notes: No hardware is needed if inserted values are copies of the input sample; otherwise, a mux and single bit flip-flop are used."  block_version=8.2.02
  Payload_Preamble : Outport  OutDataType=sfix(16)  OutScaling=2^0
  PktReset : Outport  Port=2  OutDataType=sfix(16)  OutScaling=2^0
  PktDetected : Outport  Port=3  OutDataType=sfix(16)  OutScaling=2^0
  tag scope: RxPktDone=global, RxPktTiming_SampleCount=global
  wires:
  Convert1/1 -> Up Sample2/1
  Copy_Downsample/1(Q) -> Logical5/6, Disable for Interrupts/1(PktDone)
  Assert/1 -> S-R Latch1/2(R), Counter/3, S-R Latch/2(R), PktReset/1, Up Sample/1
  Disable for Interrupts/1(Det Disabled) -> Logical5/5
  Done/1 -> Copy_Downsample/1(D), Up Sample1/1
  S-R Latch1/1(Q) -> Counter/4, PktDetected/1, Logical2/1
  S-R Latch/1(Q) -> Inverter3/1, Edge Det1/1(D)
  Edge Det1/1(Q) -> Counter/1
  Convert2/1 -> Counter/2
  Logical2/1 -> S-R Latch/1(S)
  Logical6/1 -> Logical5/2
  [regRx_reqLongCorr] -> Logical6/3
  Inverter3/1 -> Logical6/2
  Relational6/1 -> Logical6/1
  Constant11/1 -> Relational6/2
  [rx_global_reset_DV2] -> Logical5/1
  Logical5/1 -> Assert/1
  Logical3/1 -> Convert1/1, Payload_Preamble/1
  Relational5/1 -> Logical3/1
  Inverter1/1 -> Logical3/2
  [rx_global_reset_DV2] -> Inverter1/1
  [regRx_PktDet_Delay] -> ASR/2
  ASR/1 -> Edge Det/1(D)
  Pkt Det/1 -> ASR/1
  Constant6/1 -> Relational5/2
  Edge Det/1(Q) -> S-R Latch1/1(S)
  Counter/1 -> Relational5/1, Relational6/1, Fine_Pkt_det/1(Samp Count), [RxPktTiming_SampleCount]
  Fine_Pkt_det/1(Det) -> Logical2/2
  Up Sample/1 -> Rx_PktDetReset/1
  Up Sample1/1 -> Rx_debug_PktDone/1, [RxPktDone]
  Up Sample2/1 -> Rx_debug_payload/1
  Logical4/1 -> Logical5/3
  [Tx_Running] -> Logical4/1
  [regRx_forceDisableOnTx] -> Logical4/2
  Ext Reset/1(Rst) -> Logical5/4
  [regRx_PktDet_LongCorr_CountLoad] -> Convert2/1
  Long CorrA/1 -> Fine_Pkt_det/2(Long CorrA)

== system ofdm_txrx_supermimo_coded/OFDM Rx MIMO/Rx Reset Logic ==
  Constant : Constant  Value=0  OutDataType=sfix(16)  OutScaling=2^0
  Convert : xbsIndex_r4/Convert  arith_type=Boolean  n_bits=8  bin_pt=6  quantization=Truncate  overflow=Wrap  latency=0  dbl_ovrd=off  pipeline=off  block_type=convert
      settings: xl_area=[0, 0, 0, 0, 0, 0, 0]  block_version=VER_STRING_GOES_HERE
  Down Sample6 : xbsIndex_r4/Down Sample  sample_ratio=2  sample_phase="Last Value of Frame  (most efficient)"  en=off  latency=1  dbl_ovrd=off  block_type=dsamp
      settings: block_version=VER_STRING_GOES_HERE
  Logical1 : xbsIndex_r4/Logical  logical_function=OR  inputs=2  en=off  latency=0  precision=Full  arith_type=Unsigned  n_bits=8  bin_pt=2  align_bp=on  dbl_ovrd=off  block_type=logical
      settings: xl_area=[1 0 0 1 0 0 0]  block_version=VER_STRING_GOES_HERE
  Rx_Reset : xbsIndex_r4/Gateway In  arith_type=Boolean  n_bits=6  bin_pt=0  quantization="Round  (unbiased: +/- Inf)"  overflow=Saturate  period=4  dbl_ovrd=off  timing_constraint=None  locs_specified=off  LOCs={}  block_type=gatewayin
      settings: infoedit="Gateway in block.  Converts inputs of type Simulink integer, double and fixed point to  Xilinx fixed point type.<P><P>Hardware notes:  In hardware these blocks become top level input ports."  xl_area=[0 0 0 0 1 0 0]  block_version=VER_STRING_GOES_HERE
  tag scope: rx_global_reset=global, rx_global_reset_DV2=global
  wires:
  [regRx_GlobalReset] -> Logical1/1
  Logical1/1 -> [rx_global_reset], Down Sample6/1
  Rx_Reset/1 -> Convert/1
  Convert/1 -> Logical1/2
  Down Sample6/1 -> [rx_global_reset_DV2]
  Constant/1 -> Rx_Reset/1

== system ofdm_txrx_supermimo_coded/OFDM Tx MIMO/IFFT ==
  Payload Done : Inport  OutDataType=sfix(16)  OutScaling=2^0
  xn_I : Inport  Port=2  OutDataType=sfix(16)  OutScaling=2^0
  xn_Q : Inport  Port=3  OutDataType=sfix(16)  OutScaling=2^0
  Start : Inport  Port=4  OutDataType=sfix(16)  OutScaling=2^0
  Pkt Done : Inport  Port=5  OutDataType=sfix(16)  OutScaling=2^0
  Constant2 : xbsIndex_r4/Constant  arith_type=Unsigned  const=0  n_bits=1  bin_pt=0  explicit_period=off  period=1  equ=P=C  opselect=C  inp2=PCIN>>17  opr=+  inp1=P  carry=CIN  dbl_ovrd=off  block_type=constant
      settings: block_version=VER_STRING_GOES_HERE
  Constant3 : xbsIndex_r4/Constant  arith_type=Boolean  const=0  n_bits=1  bin_pt=0  explicit_period=off  period=1  equ=P=C  opselect=C  inp2=PCIN>>17  opr=+  inp1=P  carry=CIN  dbl_ovrd=off  block_type=constant
      settings: block_version=VER_STRING_GOES_HERE
  Constant4 : xbsIndex_r4/Constant  arith_type=Boolean  const=1  n_bits=1  bin_pt=0  explicit_period=off  period=1  equ=P=C  opselect=C  inp2=PCIN>>17  opr=+  inp1=P  carry=CIN  dbl_ovrd=off  block_type=constant
      settings: block_version=VER_STRING_GOES_HERE
  Constant6 : xbsIndex_r4/Constant  arith_type=Boolean  const=1  n_bits=1  bin_pt=0  explicit_period=off  period=1  equ=P=C  opselect=C  inp2=PCIN>>17  opr=+  inp1=P  carry=CIN  dbl_ovrd=off  block_type=constant
      settings: block_version=VER_STRING_GOES_HERE
  Counter : xbsIndex_r4/Counter  cnt_type=Free Running  cnt_to=Inf  operation=Up/Down  start_count=0  cnt_by_val=1  arith_type=Unsigned  n_bits=3  bin_pt=0  load_pin=off  rst=on  en=on  explicit_period=off  period=1  dbl_ovrd=off  use_behavioral_HDL=off  use_rpm=off  implementation=Fabric  block_type=counter
  "Fast Fourier Transform 6.0 " : xbsIndex_r4/Fast Fourier Transform 6.0   transform_length=64  implementation_options=pipelined_streaming_io  run_time_configurable_transform_length=off  phase_factor_width=24  scaling_options=scaled  rounding_modes=convergent_rounding  output_ordering=bit_reversed_order  cyclic_prefix_insertion=off  en=off  rst=on  ovflo=on  memory_options_data=block_ram  memory_options_phase_factors=block_ram  number_of_stages_using_block_ram_for_data_and_phase_factors=1  memory_options_reorder=block_ram  memory_options_hybrid=off  optimize_for_speed_using_xtreme_dsp_slices=off  fast_complex_mult=off  fast_butterfly=off  target_clock_frequency=250  simulation_type=external_xfix  simulation_model=fftv6_CModel:fftv6_cmodel  ip_name=Fast Fourier Transform  ip_version=6.0  dsptool_ready=true  input_ordering=natural_order  ce=true  sclr=0  wrapper_available=true  port_translation_map={ 'ce' => 'en', 'sclr' => 'rst' }  ipcore_fpga_part=xlipgetpartsetting(gcb, {'virtex4', 'xc4vsx35', '-10', 'ff668'})  target_data_throughput=50  channels=1  input_width=16  block_type=xfft_v6_0
      settings: block_version=10.1.3
  IFFT : Scope
      settings: TimeRange=392.7801724137931  YMin=-0.5  YMax=0.5  SaveName=ScopeData19
  Inverter3 : xbsIndex_r4/Inverter  en=off  latency=0  dbl_ovrd=off  block_type=inv
      settings: block_version=VER_STRING_GOES_HERE
  Logical1 : xbsIndex_r4/Logical  logical_function=AND  inputs=2  en=off  latency=0  precision=Full  arith_type=Unsigned  n_bits=16  bin_pt=0  align_bp=on  dbl_ovrd=off  block_type=logical
      settings: block_version=VER_STRING_GOES_HERE
  Logical2 : xbsIndex_r4/Logical  logical_function=XOR  inputs=2  en=off  latency=0  precision=Full  arith_type=Unsigned  n_bits=16  bin_pt=0  align_bp=on  dbl_ovrd=off  block_type=logical
      settings: block_version=VER_STRING_GOES_HERE
  Logical3 : xbsIndex_r4/Logical  logical_function=AND  inputs=2  en=off  latency=0  precision=Full  arith_type=Unsigned  n_bits=16  bin_pt=0  align_bp=on  dbl_ovrd=off  block_type=logical
      settings: block_version=VER_STRING_GOES_HERE
  Logical4 : xbsIndex_r4/Logical  logical_function=OR  inputs=2  en=off  latency=0  precision=Full  arith_type=Unsigned  n_bits=16  bin_pt=0  align_bp=on  dbl_ovrd=off  block_type=logical
      settings: block_version=VER_STRING_GOES_HERE
  Posedge2 : Subsystem
  Relational2 : xbsIndex_r4/Relational  mode=a=b  en=off  latency=0  dbl_ovrd=off  block_type=relational
      settings: xl_area=[2 0 0 4 0 0 0]  block_version=VER_STRING_GOES_HERE
  SR Latch : Subsystem
  done{1..8} (8 alike) : xbsIndex_r4/Gateway Out  hdl_port=off  timing_constraint=None  locs_specified=off  LOCs={}  block_type=gatewayout
      settings: infoedit="Gateway out block.  Converts Xilinx fixed point inputs into ouputs of type Simulink integer, double, or fixed point.<P><P>Hardware notes:  In hardware these blocks become top level output ports or are discarded, depending on how they are configured."  xl_area=[0 0 0 0 1 0 0]  block_version=VER_STRING_GOES_HERE
  xn_Index : Outport  OutDataType=sfix(16)  OutScaling=2^0
  Vout : Outport  Port=2  OutDataType=sfix(16)  OutScaling=2^0
  xk_I : Outport  Port=3  OutDataType=sfix(16)  OutScaling=2^0
  xk_Q : Outport  Port=4  OutDataType=sfix(16)  OutScaling=2^0
  xk_Index : Outport  Port=5  OutDataType=sfix(16)  OutScaling=2^0
  LastSym : Outport  Port=6  OutDataType=sfix(16)  OutScaling=2^0
  wires:
  Constant3/1 -> "Fast Fourier Transform 6.0 "/4
  Constant4/1 -> "Fast Fourier Transform 6.0 "/5
  Constant6/1 -> "Fast Fourier Transform 6.0 "/7
  Logical4/1 -> "Fast Fourier Transform 6.0 "/8, Counter/2, SR Latch/2(R)
  xn_I/1 -> "Fast Fourier Transform 6.0 "/1, done4/1
  "Fast Fourier Transform 6.0 "/1 -> xk_I/1, done1/1
  "Fast Fourier Transform 6.0 "/2 -> xk_Q/1, done2/1
  xn_Q/1 -> "Fast Fourier Transform 6.0 "/2, done5/1
  "Fast Fourier Transform 6.0 "/3 -> xn_Index/1, done3/1
  Logical1/1 -> Counter/1, Logical2/1
  "Fast Fourier Transform 6.0 "/4 -> xk_Index/1, done7/1
  "Fast Fourier Transform 6.0 "/7 -> Vout/1
  [Tx_FFTScaling] -> "Fast Fourier Transform 6.0 "/6
  "Fast Fourier Transform 6.0 "/8 -> Logical2/2
  Pkt Done/1 -> Logical4/2
  Inverter3/1 -> Logical1/2
  Start/1 -> Logical1/1, "Fast Fourier Transform 6.0 "/3, done6/1
  Logical2/1 -> Counter/3
  Constant2/1 -> Relational2/2
  Counter/1 -> Relational2/1
  Payload Done/1 -> Inverter3/1, Logical3/2
  Relational2/1 -> Logical3/1
  Logical3/1 -> Posedge2/1(D)
  SR Latch/1(Q) -> LastSym/1
  Posedge2/1(Q) -> SR Latch/1(S)
  [TxReset] -> Logical4/1
  done4/1 -> ?
  done5/1 -> ?
  done3/1 -> ?
  done6/1 -> ?
  done1/1 -> ?
  done2/1 -> ?
  done7/1 -> ?
  "Fast Fourier Transform 6.0 "/10 -> done8/1
  done8/1 -> IFFT/1
